simulink model "cddEcuMApp"  (R2022b)
settings: Solver=FixedStepDiscrete  StartTime=0.0  StopTime=10.0  FixedStep=0.02  RelTol=1e-3
undefined symbols (not defined in the file or in its folder): DioChannelType, HFAChlType, IcuChannelType, P_DioLevelType, P_Std_ReturnType, VGAChlType
settings Model: Creator=blue.ge  LastModifiedBy=blue.ge
settings DataIOCC: SaveFormat=StructureWithTime
settings OptimizationCC: DefaultParameterBehavior=Inlined  EnhancedBackFolding=on  PassReuseOutputArgsAs=Individual arguments  ZeroExternalMemoryAtStartup=off  ZeroInternalMemoryAtStartup=off  OptimizeBlockOrder=speed  DifferentSizesBufferReuse=on  OptimizationCustomize=off  EfficientTunableParamExpr=on
settings DebuggingCC: ParameterTunabilityLossMsg=error
settings ModelReferenceCC: ModelReferenceNumInstancesAllowed=Single
settings SFSimCC: MATLABDynamicMemAlloc=off
settings RTWCC: Description=AUTOSAR  SystemTargetFile=autosar.tlc  GenCodeOnly=on  TemplateMakefile=ert_default_tmf
settings CodeAppCC: ForceParamTrailComments=on  IgnoreCustomStorageClasses=off  OperatorAnnotations=on  SimulinkDataObjDesc=on  SFDataObjDesc=on  InsertBlockDesc=on
settings STFCustomTargetCC: TargetLangStandard=C99 (ISO)  MultiwordLength=256  ParMdlRefBuildCompliant=on  CompOptLevelCompliant=on  IncludeMdlTerminateFcn=off  CombineOutputUpdateFcns=on  SuppressErrorStatus=on  ERTCustomFileBanners=on  SupportNonFinite=off  SupportComplex=off  SupportContinuousTime=off  SupportNonInlinedSFcns=off  RemoveResetFunc=on  MATLABClassNameForMDSCustomization=Simulink.SoftwareTarget.ERTCustomization  AutosarCompliant=on  UseToolchainInfoCompliant=on  LookupTableObjectStructAxisOrder=2,1,3,4,...  LUTObjectStructOrderEvenSpacing=Size,Table,Breakpoints  SystemTargetFile=autosar.tlc
settings ERTTargetCC: TargetLangStandard=C99 (ISO)  ConcurrentExecutionCompliant=off  IncludeMdlTerminateFcn=off  SuppressErrorStatus=on  SupportNonFinite=off  SupportComplex=off  ModelStepFunctionPrototypeControlCompliant=off  CPPClassGenCompliant=off  AutosarCompliant=on  LookupTableObjectStructAxisOrder=2,1,3,4,...  LUTObjectStructOrderEvenSpacing=Size,Table,Breakpoints  InlinedParameterPlacement=NonHierarchical  ERTCustomFileTemplate=example_file_process.tlc

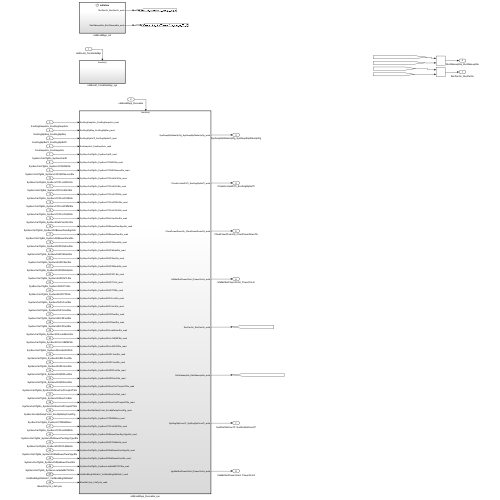
[thumbnail: Simulink's stored preview of the top-level diagram]
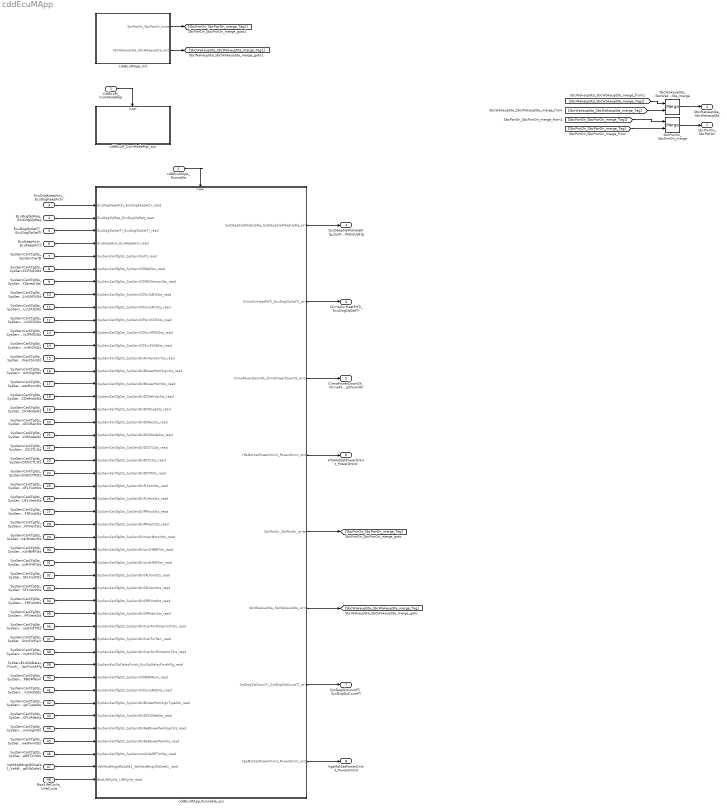
== system cddEcuMApp ==
  cddEcuM_ComModeMgr : Inport  SampleTime=0.02  OutputFunctionCall=on
  cddEcuMApp_Runnable : Inport  Port=2  SampleTime=0.02  OutputFunctionCall=on
  EcuDogKeepActv_EcuDogKeepActv : Inport  Port=3  OutDataTypeStr=Boolean  PortDimensions=1
  EcuDogSlpReq_EcuDogSlpReq : Inport  Port=4  OutDataTypeStr=Boolean  PortDimensions=1
  EcuDogSlpSetTi_EcuDogSlpSetTi : Inport  Port=5  OutDataTypeStr=Ti33  PortDimensions=1
  EcuKeepActv_EcuKeepActv : Inport  Port=6  OutDataTypeStr=Enum: OnOff1  PortDimensions=1
  SysServCarCfgSts_SysServCarID : Inport  Port=7  OutDataTypeStr=Enum: SysServCarID  PortDimensions=1
  SysServCarCfgSts_SysServCCPAQSSts : Inport  Port=8  OutDataTypeStr=UInt8  PortDimensions=1
  SysServCarCfgSts_SysServCCPHEXSensorSts : Inport  Port=9  OutDataTypeStr=UInt8  PortDimensions=1
  SysServCarCfgSts_SysServCCPLin2AFUSts : Inport  Port=10  OutDataTypeStr=Boolean  PortDimensions=1
  SysServCarCfgSts_SysServCCPLin2AIUSts : Inport  Port=11  OutDataTypeStr=UInt8  PortDimensions=1
  SysServCarCfgSts_SysServCCPLin2CDSSts : Inport  Port=12  OutDataTypeStr=Boolean  PortDimensions=1
  SysServCarCfgSts_SysServCCPLin2PMSISts : Inport  Port=13  OutDataTypeStr=Boolean  PortDimensions=1
  SysServCarCfgSts_SysServCCPLin3VGASts : Inport  Port=14  OutDataTypeStr=Boolean  PortDimensions=1
  SysServCarCfgSts_SysServDUAirVentSnrSts : Inport  Port=15  OutDataTypeStr=Boolean  PortDimensions=1
  SysServCarCfgSts_SysServDUBlowerPwmSignSts : Inport  Port=16  OutDataTypeStr=Boolean  PortDimensions=1
  SysServCarCfgSts_SysServDUBlowerPwmSts : Inport  Port=17  OutDataTypeStr=Boolean  PortDimensions=1
  SysServCarCfgSts_SysServDUDCDefrostSts : Inport  Port=18  OutDataTypeStr=UInt8  PortDimensions=1
  SysServCarCfgSts_SysServDUDCModeSts : Inport  Port=19  OutDataTypeStr=UInt8  PortDimensions=1
  SysServCarCfgSts_SysServDUDCRecSts : Inport  Port=20  OutDataTypeStr=UInt8  PortDimensions=1
  SysServCarCfgSts_SysServDUDCSModeSts : Inport  Port=21  OutDataTypeStr=UInt8  PortDimensions=1
  SysServCarCfgSts_SysServDUDCSTLSts : Inport  Port=22  OutDataTypeStr=UInt8  PortDimensions=1
  SysServCarCfgSts_SysServDUDCTLSts : Inport  Port=23  OutDataTypeStr=UInt8  PortDimensions=1
  SysServCarCfgSts_SysServDUDCTRSts : Inport  Port=24  OutDataTypeStr=UInt8  PortDimensions=1
  SysServCarCfgSts_SysServDUFLFootSts : Inport  Port=25  OutDataTypeStr=UInt8  PortDimensions=1
  SysServCarCfgSts_SysServDUFLVentSts : Inport  Port=26  OutDataTypeStr=UInt8  PortDimensions=1
  SysServCarCfgSts_SysServDUFRFootSts : Inport  Port=27  OutDataTypeStr=UInt8  PortDimensions=1
  SysServCarCfgSts_SysServDUFRVentSts : Inport  Port=28  OutDataTypeStr=UInt8  PortDimensions=1
  SysServCarCfgSts_SysServDUIncarMotorSts : Inport  Port=29  OutDataTypeStr=Boolean  PortDimensions=1
  SysServCarCfgSts_SysServDULin1HBMFSts : Inport  Port=30  OutDataTypeStr=Boolean  PortDimensions=1
  SysServCarCfgSts_SysServDULin4HFAFSts : Inport  Port=31  OutDataTypeStr=UInt32  PortDimensions=1
  SysServCarCfgSts_SysServDUSFLFootSts : Inport  Port=32  OutDataTypeStr=UInt8  PortDimensions=1
  SysServCarCfgSts_SysServDUSFLVentSts : Inport  Port=33  OutDataTypeStr=UInt8  PortDimensions=1
  SysServCarCfgSts_SysServDUSFRFootSts : Inport  Port=34  OutDataTypeStr=UInt8  PortDimensions=1
  SysServCarCfgSts_SysServDUSFRVentSts : Inport  Port=35  OutDataTypeStr=UInt8  PortDimensions=1
  SysServCarCfgSts_SysServDUSnsrForFCmptmtTSts : Inport  Port=36  OutDataTypeStr=UInt8  PortDimensions=1
  SysServCarCfgSts_SysServDUSnsrForRair : Inport  Port=37  OutDataTypeStr=UInt8  PortDimensions=1
  SysServCarCfgSts_SysServDUSnsrForRCmptmtTSts : Inport  Port=38  OutDataTypeStr=UInt8  PortDimensions=1
  SysServEcuSlpDelayFinish_EcuSlpDelayFinishFlg : Inport  Port=39  OutDataTypeStr=UInt8  PortDimensions=1
  SysServCarCfgSts_SysServCCPBGMNum : Inport  Port=40  OutDataTypeStr=UInt8  PortDimensions=1
  SysServCarCfgSts_SysServCCPLin2AQSSts : Inport  Port=41  OutDataTypeStr=Boolean  PortDimensions=1
  SysServCarCfgSts_SysServDUBlowerPwmSignTypeSts : Inport  Port=42  OutDataTypeStr=UInt8  PortDimensions=1
  SysServCarCfgSts_SysServDUDCFLMdeSts : Inport  Port=43  OutDataTypeStr=UInt8  PortDimensions=1
  SysServCarCfgSts_SysServDUReBlowerPwmSignSts : Inport  Port=44  OutDataTypeStr=UInt8  PortDimensions=1
  SysServCarCfgSts_SysServDUReBlowerPwmSts : Inport  Port=45  OutDataTypeStr=UInt8  PortDimensions=1
  SysServCarCfgSts_SysServLowSideRETXVSts : Inport  Port=46  OutDataTypeStr=UInt8  PortDimensions=1
  VehModMngtGlbSafe1_VehModMngtGlbSafe1 : Inport  Port=47  OutDataTypeStr=Bus: VehModMngtGlbSafe1  PortDimensions=1  BusOutputAsStruct=on
  BswLifeCycle_LifeCycle : Inport  Port=48  OutDataTypeStr=Enum: CCMLifeCycleModeGroup  PortDimensions=1
  SbcPwrOn_SbcPwrOn_merge : Merge
  SbcWakeupSta_SbcWakeupSta_merge : Merge
  cddEcuMApp_Init : Subsystem
  cddEcuMApp_Runnable_sys : Subsystem
  cddEcuM_ComModeMgr_sys : Subsystem
  SbcPwrOn_SbcPwrOn : Outport  OutDataTypeStr=PwrOnff  PortDimensions=1
  SbcWakeupSta_SbcWakeupSta : Outport  Port=2  OutDataTypeStr=Enum: SbcWakeupSta  PortDimensions=1
  SysDeepSlpWakeUpFlg_SysDeepSlpWakeUpFlg : Outport  Port=3  OutDataTypeStr=Boolean  PortDimensions=1
  ClimaOvrHeatPrtTi_EcuDogSlpSetTi : Outport  Port=4  OutDataTypeStr=Ti33  PortDimensions=1
  ClimaPowerDownOk_ClimaPowerDownOk : Outport  Port=5  OutDataTypeStr=Boolean  PortDimensions=1
  HfaMotSetPowerOnInit_PowerOnInit : Outport  Port=6  OutDataTypeStr=Boolean  PortDimensions=1
  SysDogSlpCountTi_SysDogSlpCountTi : Outport  Port=7  OutDataTypeStr=Ti33  PortDimensions=1
  VgaMotSetPowerOnInit_PowerOnInit : Outport  Port=8  OutDataTypeStr=Boolean  PortDimensions=1
  wires:
  EcuDogKeepActv_EcuDogKeepActv/1 -> cddEcuMApp_Runnable_sys/1(EcuDogKeepActv_EcuDogKeepActv_read)
  EcuDogSlpReq_EcuDogSlpReq/1 -> cddEcuMApp_Runnable_sys/2(EcuDogSlpReq_EcuDogSlpReq_read)
  EcuDogSlpSetTi_EcuDogSlpSetTi/1 -> cddEcuMApp_Runnable_sys/3(EcuDogSlpSetTi_EcuDogSlpSetTi_read)
  EcuKeepActv_EcuKeepActv/1 -> cddEcuMApp_Runnable_sys/4(EcuKeepActv_EcuKeepActv_read)
  SysServCarCfgSts_SysServCarID/1 -> cddEcuMApp_Runnable_sys/5(SysServCarCfgSts_SysServCarID_read)
  SysServCarCfgSts_SysServCCPAQSSts/1 -> cddEcuMApp_Runnable_sys/6(SysServCarCfgSts_SysServCCPAQSSts_read)
  SysServCarCfgSts_SysServCCPHEXSensorSts/1 -> cddEcuMApp_Runnable_sys/7(SysServCarCfgSts_SysServCCPHEXSensorSts_read)
  SysServCarCfgSts_SysServCCPLin2AFUSts/1 -> cddEcuMApp_Runnable_sys/8(SysServCarCfgSts_SysServCCPLin2AFUSts_read)
  SysServCarCfgSts_SysServCCPLin2AIUSts/1 -> cddEcuMApp_Runnable_sys/9(SysServCarCfgSts_SysServCCPLin2AIUSts_read)
  SysServCarCfgSts_SysServCCPLin2CDSSts/1 -> cddEcuMApp_Runnable_sys/10(SysServCarCfgSts_SysServCCPLin2CDSSts_read)
  SysServCarCfgSts_SysServCCPLin2PMSISts/1 -> cddEcuMApp_Runnable_sys/11(SysServCarCfgSts_SysServCCPLin2PMSISts_read)
  SysServCarCfgSts_SysServCCPLin3VGASts/1 -> cddEcuMApp_Runnable_sys/12(SysServCarCfgSts_SysServCCPLin3VGASts_read)
  SysServCarCfgSts_SysServDUAirVentSnrSts/1 -> cddEcuMApp_Runnable_sys/13(SysServCarCfgSts_SysServDUAirVentSnrSts_read)
  SysServCarCfgSts_SysServDUBlowerPwmSignSts/1 -> cddEcuMApp_Runnable_sys/14(SysServCarCfgSts_SysServDUBlowerPwmSignSts_read)
  SysServCarCfgSts_SysServDUBlowerPwmSts/1 -> cddEcuMApp_Runnable_sys/15(SysServCarCfgSts_SysServDUBlowerPwmSts_read)
  SysServCarCfgSts_SysServDUDCDefrostSts/1 -> cddEcuMApp_Runnable_sys/16(SysServCarCfgSts_SysServDUDCDefrostSts_read)
  SysServCarCfgSts_SysServDUDCModeSts/1 -> cddEcuMApp_Runnable_sys/17(SysServCarCfgSts_SysServDUDCModeSts_read)
  SysServCarCfgSts_SysServDUDCRecSts/1 -> cddEcuMApp_Runnable_sys/18(SysServCarCfgSts_SysServDUDCRecSts_read)
  SysServCarCfgSts_SysServDUDCSModeSts/1 -> cddEcuMApp_Runnable_sys/19(SysServCarCfgSts_SysServDUDCSModeSts_read)
  SysServCarCfgSts_SysServDUDCSTLSts/1 -> cddEcuMApp_Runnable_sys/20(SysServCarCfgSts_SysServDUDCSTLSts_read)
  SysServCarCfgSts_SysServDUDCTLSts/1 -> cddEcuMApp_Runnable_sys/21(SysServCarCfgSts_SysServDUDCTLSts_read)
  SysServCarCfgSts_SysServDUDCTRSts/1 -> cddEcuMApp_Runnable_sys/22(SysServCarCfgSts_SysServDUDCTRSts_read)
  SysServCarCfgSts_SysServDUFLFootSts/1 -> cddEcuMApp_Runnable_sys/23(SysServCarCfgSts_SysServDUFLFootSts_read)
  SysServCarCfgSts_SysServDUFLVentSts/1 -> cddEcuMApp_Runnable_sys/24(SysServCarCfgSts_SysServDUFLVentSts_read)
  SysServCarCfgSts_SysServDUFRFootSts/1 -> cddEcuMApp_Runnable_sys/25(SysServCarCfgSts_SysServDUFRFootSts_read)
  SysServCarCfgSts_SysServDUFRVentSts/1 -> cddEcuMApp_Runnable_sys/26(SysServCarCfgSts_SysServDUFRVentSts_read)
  SysServCarCfgSts_SysServDUIncarMotorSts/1 -> cddEcuMApp_Runnable_sys/27(SysServCarCfgSts_SysServDUIncarMotorSts_read)
  SysServCarCfgSts_SysServDULin1HBMFSts/1 -> cddEcuMApp_Runnable_sys/28(SysServCarCfgSts_SysServDULin1HBMFSts_read)
  SysServCarCfgSts_SysServDULin4HFAFSts/1 -> cddEcuMApp_Runnable_sys/29(SysServCarCfgSts_SysServDULin4HFAFSts_read)
  SysServCarCfgSts_SysServDUSFLFootSts/1 -> cddEcuMApp_Runnable_sys/30(SysServCarCfgSts_SysServDUSFLFootSts_read)
  SysServCarCfgSts_SysServDUSFLVentSts/1 -> cddEcuMApp_Runnable_sys/31(SysServCarCfgSts_SysServDUSFLVentSts_read)
  SysServCarCfgSts_SysServDUSFRFootSts/1 -> cddEcuMApp_Runnable_sys/32(SysServCarCfgSts_SysServDUSFRFootSts_read)
  SysServCarCfgSts_SysServDUSFRVentSts/1 -> cddEcuMApp_Runnable_sys/33(SysServCarCfgSts_SysServDUSFRVentSts_read)
  SysServCarCfgSts_SysServDUSnsrForFCmptmtTSts/1 -> cddEcuMApp_Runnable_sys/34(SysServCarCfgSts_SysServDUSnsrForFCmptmtTSts_read)
  SysServCarCfgSts_SysServDUSnsrForRair/1 -> cddEcuMApp_Runnable_sys/35(SysServCarCfgSts_SysServDUSnsrForRair_read)
  SysServCarCfgSts_SysServDUSnsrForRCmptmtTSts/1 -> cddEcuMApp_Runnable_sys/36(SysServCarCfgSts_SysServDUSnsrForRCmptmtTSts_read)
  SysServEcuSlpDelayFinish_EcuSlpDelayFinishFlg/1 -> cddEcuMApp_Runnable_sys/37(SysServEcuSlpDelayFinish_EcuSlpDelayFinishFlg_read)
  SysServCarCfgSts_SysServCCPBGMNum/1 -> cddEcuMApp_Runnable_sys/38(SysServCarCfgSts_SysServCCPBGMNum_read)
  SysServCarCfgSts_SysServCCPLin2AQSSts/1 -> cddEcuMApp_Runnable_sys/39(SysServCarCfgSts_SysServCCPLin2AQSSts_read)
  SysServCarCfgSts_SysServDUBlowerPwmSignTypeSts/1 -> cddEcuMApp_Runnable_sys/40(SysServCarCfgSts_SysServDUBlowerPwmSignTypeSts_read)
  SysServCarCfgSts_SysServDUDCFLMdeSts/1 -> cddEcuMApp_Runnable_sys/41(SysServCarCfgSts_SysServDUDCFLMdeSts_read)
  SysServCarCfgSts_SysServDUReBlowerPwmSignSts/1 -> cddEcuMApp_Runnable_sys/42(SysServCarCfgSts_SysServDUReBlowerPwmSignSts_read)
  SysServCarCfgSts_SysServDUReBlowerPwmSts/1 -> cddEcuMApp_Runnable_sys/43(SysServCarCfgSts_SysServDUReBlowerPwmSts_read)
  SysServCarCfgSts_SysServLowSideRETXVSts/1 -> cddEcuMApp_Runnable_sys/44(SysServCarCfgSts_SysServLowSideRETXVSts_read)
  VehModMngtGlbSafe1_VehModMngtGlbSafe1/1 -> cddEcuMApp_Runnable_sys/45(VehModMngtGlbSafe1_VehModMngtGlbSafe1_read)
  cddEcuMApp_Runnable_sys/1(SysDeepSlpWakeUpFlg_SysDeepSlpWakeUpFlg_write) -> SysDeepSlpWakeUpFlg_SysDeepSlpWakeUpFlg/1
  cddEcuMApp_Runnable_sys/2(ClimaOvrHeatPrtTi_EcuDogSlpSetTi_write) -> ClimaOvrHeatPrtTi_EcuDogSlpSetTi/1
  cddEcuMApp_Runnable_sys/3(ClimaPowerDownOk_ClimaPowerDownOk_write) -> ClimaPowerDownOk_ClimaPowerDownOk/1
  cddEcuMApp_Runnable_sys/4(HfaMotSetPowerOnInit_PowerOnInit_write) -> HfaMotSetPowerOnInit_PowerOnInit/1
  SbcPwrOn_SbcPwrOn_merge/1 -> SbcPwrOn_SbcPwrOn/1
  SbcWakeupSta_SbcWakeupSta_merge/1 -> SbcWakeupSta_SbcWakeupSta/1
  cddEcuMApp_Runnable_sys/7(SysDogSlpCountTi_SysDogSlpCountTi_write) -> SysDogSlpCountTi_SysDogSlpCountTi/1
  cddEcuMApp_Runnable_sys/8(VgaMotSetPowerOnInit_PowerOnInit_write) -> VgaMotSetPowerOnInit_PowerOnInit/1
  BswLifeCycle_LifeCycle/1 -> cddEcuMApp_Runnable_sys/46(BswLifeCycle_LifeCycle_read)
  cddEcuMApp_Runnable_sys/6(SbcWakeupSta_SbcWakeupSta_write) -> [SbcWakeupSta_SbcWakeupSta_merge_Tag]
  [SbcWakeupSta_SbcWakeupSta_merge_Tag] -> SbcWakeupSta_SbcWakeupSta_merge/2
  cddEcuMApp_Init/2(SbcWakeupSta_SbcWakeupSta_write) -> [SbcWakeupSta_SbcWakeupSta_merge_Tag1]
  [SbcWakeupSta_SbcWakeupSta_merge_Tag1] -> SbcWakeupSta_SbcWakeupSta_merge/1
  cddEcuMApp_Runnable_sys/5(SbcPwrOn_SbcPwrOn_write) -> [SbcPwrOn_SbcPwrOn_merge_Tag]
  [SbcPwrOn_SbcPwrOn_merge_Tag] -> SbcPwrOn_SbcPwrOn_merge/2
  cddEcuMApp_Init/1(SbcPwrOn_SbcPwrOn_write) -> [SbcPwrOn_SbcPwrOn_merge_Tag1]
  [SbcPwrOn_SbcPwrOn_merge_Tag1] -> SbcPwrOn_SbcPwrOn_merge/1
  cddEcuM_ComModeMgr/1 -> cddEcuM_ComModeMgr_sys/Trigger
  cddEcuMApp_Runnable/1 -> cddEcuMApp_Runnable_sys/Trigger

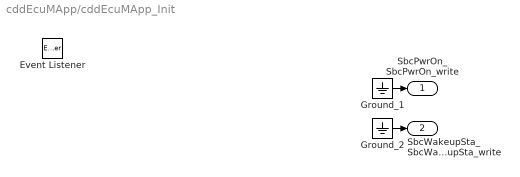
== system cddEcuMApp/cddEcuMApp_Init ==
  Event Listener : EventListener  EventName=reset
  Ground_1 : Ground
  Ground_2 : Ground
  SbcPwrOn_SbcPwrOn_write : Outport
  SbcWakeupSta_SbcWakeupSta_write : Outport  Port=2
  wires:
  Ground_1/1 -> SbcPwrOn_SbcPwrOn_write/1
  Ground_2/1 -> SbcWakeupSta_SbcWakeupSta_write/1

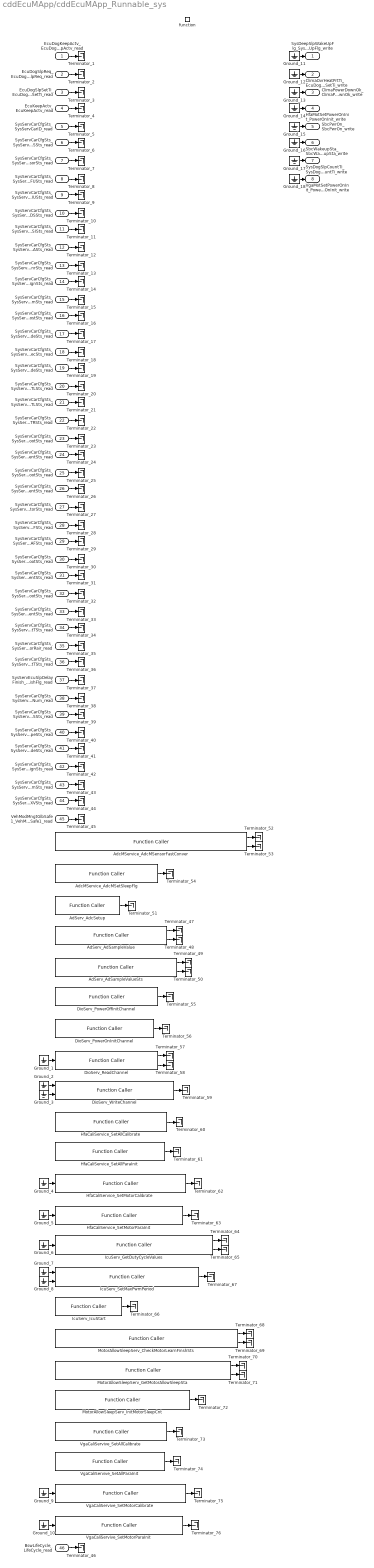
== system cddEcuMApp/cddEcuMApp_Runnable_sys ==
  EcuDogKeepActv_EcuDogKeepActv_read : Inport
  EcuDogSlpReq_EcuDogSlpReq_read : Inport  Port=2
  EcuDogSlpSetTi_EcuDogSlpSetTi_read : Inport  Port=3
  EcuKeepActv_EcuKeepActv_read : Inport  Port=4
  SysServCarCfgSts_SysServCarID_read : Inport  Port=5
  SysServCarCfgSts_SysServCCPAQSSts_read : Inport  Port=6
  SysServCarCfgSts_SysServCCPHEXSensorSts_read : Inport  Port=7
  SysServCarCfgSts_SysServCCPLin2AFUSts_read : Inport  Port=8
  SysServCarCfgSts_SysServCCPLin2AIUSts_read : Inport  Port=9
  SysServCarCfgSts_SysServCCPLin2CDSSts_read : Inport  Port=10
  SysServCarCfgSts_SysServCCPLin2PMSISts_read : Inport  Port=11
  SysServCarCfgSts_SysServCCPLin3VGASts_read : Inport  Port=12
  SysServCarCfgSts_SysServDUAirVentSnrSts_read : Inport  Port=13
  SysServCarCfgSts_SysServDUBlowerPwmSignSts_read : Inport  Port=14
  SysServCarCfgSts_SysServDUBlowerPwmSts_read : Inport  Port=15
  SysServCarCfgSts_SysServDUDCDefrostSts_read : Inport  Port=16
  SysServCarCfgSts_SysServDUDCModeSts_read : Inport  Port=17
  SysServCarCfgSts_SysServDUDCRecSts_read : Inport  Port=18
  SysServCarCfgSts_SysServDUDCSModeSts_read : Inport  Port=19
  SysServCarCfgSts_SysServDUDCSTLSts_read : Inport  Port=20
  SysServCarCfgSts_SysServDUDCTLSts_read : Inport  Port=21
  SysServCarCfgSts_SysServDUDCTRSts_read : Inport  Port=22
  SysServCarCfgSts_SysServDUFLFootSts_read : Inport  Port=23
  SysServCarCfgSts_SysServDUFLVentSts_read : Inport  Port=24
  SysServCarCfgSts_SysServDUFRFootSts_read : Inport  Port=25
  SysServCarCfgSts_SysServDUFRVentSts_read : Inport  Port=26
  SysServCarCfgSts_SysServDUIncarMotorSts_read : Inport  Port=27
  SysServCarCfgSts_SysServDULin1HBMFSts_read : Inport  Port=28
  SysServCarCfgSts_SysServDULin4HFAFSts_read : Inport  Port=29
  SysServCarCfgSts_SysServDUSFLFootSts_read : Inport  Port=30
  SysServCarCfgSts_SysServDUSFLVentSts_read : Inport  Port=31
  SysServCarCfgSts_SysServDUSFRFootSts_read : Inport  Port=32
  SysServCarCfgSts_SysServDUSFRVentSts_read : Inport  Port=33
  SysServCarCfgSts_SysServDUSnsrForFCmptmtTSts_read : Inport  Port=34
  SysServCarCfgSts_SysServDUSnsrForRair_read : Inport  Port=35
  SysServCarCfgSts_SysServDUSnsrForRCmptmtTSts_read : Inport  Port=36
  SysServEcuSlpDelayFinish_EcuSlpDelayFinishFlg_read : Inport  Port=37
  SysServCarCfgSts_SysServCCPBGMNum_read : Inport  Port=38
  SysServCarCfgSts_SysServCCPLin2AQSSts_read : Inport  Port=39
  SysServCarCfgSts_SysServDUBlowerPwmSignTypeSts_read : Inport  Port=40
  SysServCarCfgSts_SysServDUDCFLMdeSts_read : Inport  Port=41
  SysServCarCfgSts_SysServDUReBlowerPwmSignSts_read : Inport  Port=42
  SysServCarCfgSts_SysServDUReBlowerPwmSts_read : Inport  Port=43
  SysServCarCfgSts_SysServLowSideRETXVSts_read : Inport  Port=44
  VehModMngtGlbSafe1_VehModMngtGlbSafe1_read : Inport  Port=45
  BswLifeCycle_LifeCycle_read : Inport  Port=46
  function : TriggerPort  TriggerType=function-call  VariantControl=(inherit)  SampleTimeType=periodic  SampleTime=-1
  AdServ_AdSampleValue : FunctionCaller  FunctionPrototype=[AdValue,ERR] = AdServ_AdSampleValue()  OutputArgumentSpecifications=P_SampleValueType, P_Std_ReturnType
  AdServ_AdSampleValueSts : FunctionCaller  FunctionPrototype=[AdValue,ERR] = AdServ_AdSampleValueSts()  OutputArgumentSpecifications=P_SampleValueTypeSts, P_Std_ReturnType
  AdServ_AdcSetup : FunctionCaller  FunctionPrototype=ERR = AdServ_AdcSetup()  OutputArgumentSpecifications=P_Std_ReturnType
  AdcMService_AdcMSensorFastConver : FunctionCaller  FunctionPrototype=[SensorFastConverFlag,ERR] = AdcMService_AdcMSensorFastConver()  OutputArgumentSpecifications=P_AdcMSensorFastConver, P_Std_ReturnType
  AdcMService_AdcMSetSleepFlg : FunctionCaller  FunctionPrototype=ERR = AdcMService_AdcMSetSleepFlg()  OutputArgumentSpecifications=P_Std_ReturnType
  DioServ_PowerOffInitChannel : FunctionCaller  FunctionPrototype=ERR = DioServ_PowerOffInitChannel()  OutputArgumentSpecifications=P_Std_ReturnType
  DioServ_PowerOnInitChannel : FunctionCaller  FunctionPrototype=ERR = DioServ_PowerOnInitChannel()  OutputArgumentSpecifications=P_Std_ReturnType
  DioServ_ReadChannel : FunctionCaller  FunctionPrototype=[Level,ERR] = DioServ_ReadChannel(ChannelId)  InputArgumentSpecifications=DioChannelType(0)  OutputArgumentSpecifications=P_DioLevelType, P_Std_ReturnType
  DioServ_WriteChannel : FunctionCaller  FunctionPrototype=ERR = DioServ_WriteChannel(ChannelId,Level)  InputArgumentSpecifications=DioChannelType(0), P_DioLevelType  OutputArgumentSpecifications=P_Std_ReturnType
  Ground_{1..18} (18 alike) : Ground
  HfaCaliService_SetAllCalibrate : FunctionCaller  FunctionPrototype=ERR = HfaCaliService_SetAllCalibrate()  OutputArgumentSpecifications=P_Std_ReturnType
  HfaCaliService_SetAllParaInit : FunctionCaller  FunctionPrototype=ERR = HfaCaliService_SetAllParaInit()  OutputArgumentSpecifications=P_Std_ReturnType
  HfaCaliService_SetMotorCalibrate : FunctionCaller  FunctionPrototype=ERR = HfaCaliService_SetMotorCalibrate(Channel)  InputArgumentSpecifications=HFAChlType(0)  OutputArgumentSpecifications=P_Std_ReturnType
  HfaCaliService_SetMotorParaInit : FunctionCaller  FunctionPrototype=ERR = HfaCaliService_SetMotorParaInit(Channel)  InputArgumentSpecifications=HFAChlType(0)  OutputArgumentSpecifications=P_Std_ReturnType
  IcuServ_GetDutyCycleValues : FunctionCaller  FunctionPrototype=[DutyCycleValues,ERR] = IcuServ_GetDutyCycleValues(Channel)  InputArgumentSpecifications=IcuChannelType(0)  OutputArgumentSpecifications=P_IcuDutyCycleType, P_Std_ReturnType
  IcuServ_IcuStart : FunctionCaller  FunctionPrototype=ERR = IcuServ_IcuStart()  OutputArgumentSpecifications=P_Std_ReturnType
  IcuServ_SetMaxPwmPeriod : FunctionCaller  FunctionPrototype=ERR = IcuServ_SetMaxPwmPeriod(Channel,MaxPwmPeriod)  InputArgumentSpecifications=IcuChannelType(0), uint16(1)  OutputArgumentSpecifications=P_Std_ReturnType
  MotorAllowSleepServ_CheckMotorLearnFinshSts : FunctionCaller  FunctionPrototype=[Status,ERR] = MotorAllowSleepServ_CheckMotorLearnFinshSts()  OutputArgumentSpecifications=uint8(1), P_Std_ReturnType
  MotorAllowSleepServ_GetMotorAllowSleepSta : FunctionCaller  FunctionPrototype=[Status,ERR] = MotorAllowSleepServ_GetMotorAllowSleepSta()  OutputArgumentSpecifications=uint8(1), P_Std_ReturnType
  MotorAllowSleepServ_InitMotorSleepCnt : FunctionCaller  FunctionPrototype=ERR = MotorAllowSleepServ_InitMotorSleepCnt()  OutputArgumentSpecifications=P_Std_ReturnType
  Terminator_{1..76} (76 alike) : Terminator
  VgaCaliServive_SetAllCalibrate : FunctionCaller  FunctionPrototype=ERR = VgaCaliServive_SetAllCalibrate()  OutputArgumentSpecifications=P_Std_ReturnType
  VgaCaliServive_SetAllParaInit : FunctionCaller  FunctionPrototype=ERR = VgaCaliServive_SetAllParaInit()  OutputArgumentSpecifications=P_Std_ReturnType
  VgaCaliServive_SetMotorCalibrate : FunctionCaller  FunctionPrototype=ERR = VgaCaliServive_SetMotorCalibrate(Channel)  InputArgumentSpecifications=VGAChlType(0)  OutputArgumentSpecifications=P_Std_ReturnType
  VgaCaliServive_SetMotorParaInit : FunctionCaller  FunctionPrototype=ERR = VgaCaliServive_SetMotorParaInit(Channel)  InputArgumentSpecifications=VGAChlType(0)  OutputArgumentSpecifications=P_Std_ReturnType
  SysDeepSlpWakeUpFlg_SysDeepSlpWakeUpFlg_write : Outport
      settings: EnsureOutportIsVirtual=on
  ClimaOvrHeatPrtTi_EcuDogSlpSetTi_write : Outport  Port=2
      settings: EnsureOutportIsVirtual=on
  ClimaPowerDownOk_ClimaPowerDownOk_write : Outport  Port=3
      settings: EnsureOutportIsVirtual=on
  HfaMotSetPowerOnInit_PowerOnInit_write : Outport  Port=4
      settings: EnsureOutportIsVirtual=on
  SbcPwrOn_SbcPwrOn_write : Outport  Port=5
      settings: EnsureOutportIsVirtual=on
  SbcWakeupSta_SbcWakeupSta_write : Outport  Port=6
      settings: EnsureOutportIsVirtual=on
  SysDogSlpCountTi_SysDogSlpCountTi_write : Outport  Port=7
      settings: EnsureOutportIsVirtual=on
  VgaMotSetPowerOnInit_PowerOnInit_write : Outport  Port=8
      settings: EnsureOutportIsVirtual=on
  wires:
  EcuDogKeepActv_EcuDogKeepActv_read/1 -> Terminator_1/1
  EcuDogSlpReq_EcuDogSlpReq_read/1 -> Terminator_2/1
  EcuDogSlpSetTi_EcuDogSlpSetTi_read/1 -> Terminator_3/1
  EcuKeepActv_EcuKeepActv_read/1 -> Terminator_4/1
  SysServCarCfgSts_SysServCarID_read/1 -> Terminator_5/1
  SysServCarCfgSts_SysServCCPAQSSts_read/1 -> Terminator_6/1
  SysServCarCfgSts_SysServCCPHEXSensorSts_read/1 -> Terminator_7/1
  SysServCarCfgSts_SysServCCPLin2AFUSts_read/1 -> Terminator_8/1
  SysServCarCfgSts_SysServCCPLin2AIUSts_read/1 -> Terminator_9/1
  SysServCarCfgSts_SysServCCPLin2CDSSts_read/1 -> Terminator_10/1
  SysServCarCfgSts_SysServCCPLin2PMSISts_read/1 -> Terminator_11/1
  SysServCarCfgSts_SysServCCPLin3VGASts_read/1 -> Terminator_12/1
  SysServCarCfgSts_SysServDUAirVentSnrSts_read/1 -> Terminator_13/1
  SysServCarCfgSts_SysServDUBlowerPwmSignSts_read/1 -> Terminator_14/1
  SysServCarCfgSts_SysServDUBlowerPwmSts_read/1 -> Terminator_15/1
  SysServCarCfgSts_SysServDUDCDefrostSts_read/1 -> Terminator_16/1
  SysServCarCfgSts_SysServDUDCModeSts_read/1 -> Terminator_17/1
  SysServCarCfgSts_SysServDUDCRecSts_read/1 -> Terminator_18/1
  SysServCarCfgSts_SysServDUDCSModeSts_read/1 -> Terminator_19/1
  SysServCarCfgSts_SysServDUDCSTLSts_read/1 -> Terminator_20/1
  SysServCarCfgSts_SysServDUDCTLSts_read/1 -> Terminator_21/1
  SysServCarCfgSts_SysServDUDCTRSts_read/1 -> Terminator_22/1
  SysServCarCfgSts_SysServDUFLFootSts_read/1 -> Terminator_23/1
  SysServCarCfgSts_SysServDUFLVentSts_read/1 -> Terminator_24/1
  SysServCarCfgSts_SysServDUFRFootSts_read/1 -> Terminator_25/1
  SysServCarCfgSts_SysServDUFRVentSts_read/1 -> Terminator_26/1
  SysServCarCfgSts_SysServDUIncarMotorSts_read/1 -> Terminator_27/1
  SysServCarCfgSts_SysServDULin1HBMFSts_read/1 -> Terminator_28/1
  SysServCarCfgSts_SysServDULin4HFAFSts_read/1 -> Terminator_29/1
  SysServCarCfgSts_SysServDUSFLFootSts_read/1 -> Terminator_30/1
  SysServCarCfgSts_SysServDUSFLVentSts_read/1 -> Terminator_31/1
  SysServCarCfgSts_SysServDUSFRFootSts_read/1 -> Terminator_32/1
  SysServCarCfgSts_SysServDUSFRVentSts_read/1 -> Terminator_33/1
  SysServCarCfgSts_SysServDUSnsrForFCmptmtTSts_read/1 -> Terminator_34/1
  SysServCarCfgSts_SysServDUSnsrForRair_read/1 -> Terminator_35/1
  SysServCarCfgSts_SysServDUSnsrForRCmptmtTSts_read/1 -> Terminator_36/1
  SysServEcuSlpDelayFinish_EcuSlpDelayFinishFlg_read/1 -> Terminator_37/1
  SysServCarCfgSts_SysServCCPBGMNum_read/1 -> Terminator_38/1
  SysServCarCfgSts_SysServCCPLin2AQSSts_read/1 -> Terminator_39/1
  SysServCarCfgSts_SysServDUBlowerPwmSignTypeSts_read/1 -> Terminator_40/1
  SysServCarCfgSts_SysServDUDCFLMdeSts_read/1 -> Terminator_41/1
  SysServCarCfgSts_SysServDUReBlowerPwmSignSts_read/1 -> Terminator_42/1
  SysServCarCfgSts_SysServDUReBlowerPwmSts_read/1 -> Terminator_43/1
  SysServCarCfgSts_SysServLowSideRETXVSts_read/1 -> Terminator_44/1
  VehModMngtGlbSafe1_VehModMngtGlbSafe1_read/1 -> Terminator_45/1
  BswLifeCycle_LifeCycle_read/1 -> Terminator_46/1
  AdServ_AdSampleValue/1 -> Terminator_47/1
  AdServ_AdSampleValue/2 -> Terminator_48/1
  AdServ_AdSampleValueSts/1 -> Terminator_49/1
  AdServ_AdSampleValueSts/2 -> Terminator_50/1
  AdServ_AdcSetup/1 -> Terminator_51/1
  AdcMService_AdcMSensorFastConver/1 -> Terminator_52/1
  AdcMService_AdcMSensorFastConver/2 -> Terminator_53/1
  AdcMService_AdcMSetSleepFlg/1 -> Terminator_54/1
  DioServ_PowerOffInitChannel/1 -> Terminator_55/1
  DioServ_PowerOnInitChannel/1 -> Terminator_56/1
  Ground_1/1 -> DioServ_ReadChannel/1
  DioServ_ReadChannel/1 -> Terminator_57/1
  DioServ_ReadChannel/2 -> Terminator_58/1
  Ground_2/1 -> DioServ_WriteChannel/1
  Ground_3/1 -> DioServ_WriteChannel/2
  DioServ_WriteChannel/1 -> Terminator_59/1
  HfaCaliService_SetAllCalibrate/1 -> Terminator_60/1
  HfaCaliService_SetAllParaInit/1 -> Terminator_61/1
  Ground_4/1 -> HfaCaliService_SetMotorCalibrate/1
  HfaCaliService_SetMotorCalibrate/1 -> Terminator_62/1
  Ground_5/1 -> HfaCaliService_SetMotorParaInit/1
  HfaCaliService_SetMotorParaInit/1 -> Terminator_63/1
  Ground_6/1 -> IcuServ_GetDutyCycleValues/1
  IcuServ_GetDutyCycleValues/1 -> Terminator_64/1
  IcuServ_GetDutyCycleValues/2 -> Terminator_65/1
  IcuServ_IcuStart/1 -> Terminator_66/1
  Ground_7/1 -> IcuServ_SetMaxPwmPeriod/1
  Ground_8/1 -> IcuServ_SetMaxPwmPeriod/2
  IcuServ_SetMaxPwmPeriod/1 -> Terminator_67/1
  MotorAllowSleepServ_CheckMotorLearnFinshSts/1 -> Terminator_68/1
  MotorAllowSleepServ_CheckMotorLearnFinshSts/2 -> Terminator_69/1
  MotorAllowSleepServ_GetMotorAllowSleepSta/1 -> Terminator_70/1
  MotorAllowSleepServ_GetMotorAllowSleepSta/2 -> Terminator_71/1
  MotorAllowSleepServ_InitMotorSleepCnt/1 -> Terminator_72/1
  VgaCaliServive_SetAllCalibrate/1 -> Terminator_73/1
  VgaCaliServive_SetAllParaInit/1 -> Terminator_74/1
  Ground_9/1 -> VgaCaliServive_SetMotorCalibrate/1
  VgaCaliServive_SetMotorCalibrate/1 -> Terminator_75/1
  Ground_10/1 -> VgaCaliServive_SetMotorParaInit/1
  VgaCaliServive_SetMotorParaInit/1 -> Terminator_76/1
  Ground_11/1 -> SysDeepSlpWakeUpFlg_SysDeepSlpWakeUpFlg_write/1
  Ground_12/1 -> ClimaOvrHeatPrtTi_EcuDogSlpSetTi_write/1
  Ground_13/1 -> ClimaPowerDownOk_ClimaPowerDownOk_write/1
  Ground_14/1 -> HfaMotSetPowerOnInit_PowerOnInit_write/1
  Ground_15/1 -> SbcPwrOn_SbcPwrOn_write/1
  Ground_16/1 -> SbcWakeupSta_SbcWakeupSta_write/1
  Ground_17/1 -> SysDogSlpCountTi_SysDogSlpCountTi_write/1
  Ground_18/1 -> VgaMotSetPowerOnInit_PowerOnInit_write/1

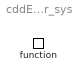
== system cddEcuMApp/cddEcuM_ComModeMgr_sys ==
  function : TriggerPort  TriggerType=function-call  VariantControl=(inherit)  SampleTimeType=periodic  SampleTime=-1
